simulink model "AcasXuClosedLoop_all_comp2017b"  (Simulink 9.0)
settings: Solver=VariableStepAuto  StartTime=0.0  StopTime=20  RelTol=1e-3
libraries: neural x14040, simulink x2
(part 30 of 534)

== system AcasXuClosedLoop_all_comp2017b/networks/Networks_m_7/AcasXu_3_7/Layer 3 ==
  "a{2} " : Inport  PortDimensions=50
      mask Display: e = 0.06;c1 = [120 230 180]/255;c2 = ([120 230 180]+0)/(256+128);c3 = ([120 230 180]+128)/(256+128);patch([0 1 1 0],[0 0 1 1],c1);patch([0 1 1 1-e 1-e 0+e],[0 0 1 1-e 0+e 0+e],c2);patch([0 0 1 1-e 0+e 0+e],[0 1 1 1-e 1-e 0+e],c3);plot([0 1 1 0 0],[0 0 1 1 0]);
  Delays 1 : Subsystem
      mask Display: e = 0.06;c1 = [120 150 230]/255;c2 = ([120 150 230]+0)/383;c3 = ([120 150 230]+128)/383;patch([0 1 1 0],[0 0 1 1],c1);patch([0 1 1 1-e 1-e 0+e],[0 0 1 1-e 0+e 0+e],c2);patch([0 0 1 1-e 0+e 0+e],[0 1 1 1-e 1-e 0+e],c3);plot([0 1 1 0 0],[0 0 1 1 0]);disp('0');
  "LW{3,2}" : Subsystem
      mask Display: e = 0.06;c1 = [120 150 230]/255;c2 = ([120 150 230]+0)/383;c3 = ([120 150 230]+128)/383;patch([0 1 1 0],[0 0 1 1],c1);patch([0 1 1 1-e 1-e 0+e],[0 0 1 1-e 0+e 0+e],c2);patch([0 0 1 1-e 0+e 0+e],[0 1 1 1-e 1-e 0+e],c3);plot([0 1 1 0 0],[0 0 1 1 0]);disp('W');
  "b{3}" : Constant  Value=[-0.5160109999999999974562570059788413345813751220703125;-1.036350000000000104449782156734727323055267333984375;-0.1930449999999999943778306032982072792947292327880859375;-1.3833800000000000540723021913436241447925567626953125;-0.9235130000000000283222334473975934088230133056640625;-1.2440800000000000746780415283865295350551605224609375;0.7431229999999999780158077555825002491474151611328125;-0.64134700000000000041922021409845910966396331787109375;-1.583579999999999987636556397774256765842437744140625;-0.76183800000000001517719283583573997020721435546875;-0.2049209999999999920472504300050786696374416351318359375;-1.2468999999999998973265746826655231416225433349609375;-0.497601000000000015521806062679388560354709625244140625;-1.144530000000000047322146201622672379016876220703125;1.0850699999999999789679350215010344982147216796875;-0.288870000000000015649703755116206593811511993408203125;-2.07702000000000008839151632855646312236785888671875;-0.65424599999999999422328755827038548886775970458984375;-1.87901000000000006906475391588173806667327880859375;-1.623250000000000081712414612411521375179290771484375;-0.0298465000000000015012435738981366739608347415924072265625;-1.91349999999999997868371792719699442386627197265625;-0.6310480000000000533333377461531199514865875244140625;-0.00068311999999999995121513496343368387897498905658721923828125;-1.6211100000000000509459141539991833269596099853515625;-1.2762800000000000810729261502274312078952789306640625;-0.61060099999999994935251379502005875110626220703125;-0.5314229999999999787263504913426004350185394287109375;-0.2066989999999999938484762651569326408207416534423828125;0.50820399999999998907895815136726014316082000732421875;-1.0196799999999999197797251326846890151500701904296875;-2.289410000000000167119651450775563716888427734375;0.65037800000000001166000629382324405014514923095703125;-1.8272999999999999243271986415493302047252655029296875;-1.99828999999999989967136571067385375499725341796875;-1.2788999999999999257482841130695305764675140380859375;-2.06318000000000001392663762089796364307403564453125;-0.86935200000000001363531509923632256686687469482421875;-2.26323000000000007503331289626657962799072265625;-0.0320645999999999986584953148849308490753173828125;-0.3529809999999999892139612711616791784763336181640625;-1.2028399999999999092636926434352062642574310302734375;-0.513816999999999968196107147377915680408477783203125;-0.6795139999999999513846660192939452826976776123046875;0.144722999999999990539123473354266025125980377197265625;0.28890300000000002089706185870454646646976470947265625;-0.7935410000000000518838305652025155723094940185546875;-0.295134999999999980691001155719277448952198028564453125;0.68889999999999995683452880257391370832920074462890625;-0.305371000000000003549160965121700428426265716552734375]
      mask Display: e = 0.06;c1 = [120 150 230]/255;c2 = ([120 150 230]+0)/383;c3 = ([120 150 230]+128)/383;patch([0 1 1 0],[0 0 1 1],c1);patch([0 1 1 1-e 1-e 0+e],[0 0 1 1-e 0+e 0+e],c2);patch([0 0 1 1-e 0+e 0+e],[0 1 1 1-e 1-e 0+e],c3);plot([0 1 1 0 0],[0 0 1 1 0]);disp('b');
  netsum : Sum  Inputs=2  SaturateOnIntegerOverflow=on  InputSameDT=on  OutDataTypeStr=Inherit: Same as first input
      mask Display: e = 0.06;c1 = [120 150 230]/255;c2 = ([120 150 230]+0)/383;c3 = ([120 150 230]+128)/383;patch([0 1 1 0],[0 0 1 1],c1);patch([0 1 1 1-e 1-e 0+e],[0 0 1 1-e 0+e 0+e],c2);patch([0 0 1 1-e 0+e 0+e],[0 1 1 1-e 1-e 0+e],c3);plot([0 1 1 0 0],[0 0 1 1 0]);disp('+');
  poslin : neural/Transfer Functions/poslin
      settings: SourceProductName=Deep Learning Toolbox  SourceProductBaseCode=NN
  "a{3}" : Outport  InitialOutput=[0;0;0;0;0;0;0;0;0;0;0;0;0;0;0;0;0;0;0;0;0;0;0;0;0;0;0;0;0;0;0;0;0;0;0;0;0;0;0;0;0;0;0;0;0;0;0;0;0;0]
      mask Display: e = 0.06;c1 = [120 230 180]/255;c2 = ([120 230 180]+0)/(256+128);c3 = ([120 230 180]+128)/(256+128);patch([0 1 1 0],[0 0 1 1],c1);patch([0 1 1 1-e 1-e 0+e],[0 0 1 1-e 0+e 0+e],c2);patch([0 0 1 1-e 0+e 0+e],[0 1 1 1-e 1-e 0+e],c3);plot([0 1 1 0 0],[0 0 1 1 0]);
  wires:
  poslin/1 -> "a{3}"/1
  netsum/1 -> poslin/1
  "b{3}"/1 -> netsum/2
  "LW{3,2}"/1("lz{3,2}") -> netsum/1
  Delays 1/1("pd{3,2}") -> "LW{3,2}"/1("ad{3,2}")
  "a{2} "/1 -> Delays 1/1("p{3}")

== system AcasXuClosedLoop_all_comp2017b/networks/Networks_m_7/AcasXu_3_7/Layer 4 ==
  "a{3} " : Inport  PortDimensions=50
      mask Display: e = 0.06;c1 = [120 230 180]/255;c2 = ([120 230 180]+0)/(256+128);c3 = ([120 230 180]+128)/(256+128);patch([0 1 1 0],[0 0 1 1],c1);patch([0 1 1 1-e 1-e 0+e],[0 0 1 1-e 0+e 0+e],c2);patch([0 0 1 1-e 0+e 0+e],[0 1 1 1-e 1-e 0+e],c3);plot([0 1 1 0 0],[0 0 1 1 0]);
  Delays 1 : Subsystem
      mask Display: e = 0.06;c1 = [120 150 230]/255;c2 = ([120 150 230]+0)/383;c3 = ([120 150 230]+128)/383;patch([0 1 1 0],[0 0 1 1],c1);patch([0 1 1 1-e 1-e 0+e],[0 0 1 1-e 0+e 0+e],c2);patch([0 0 1 1-e 0+e 0+e],[0 1 1 1-e 1-e 0+e],c3);plot([0 1 1 0 0],[0 0 1 1 0]);disp('0');
  "LW{4,3}" : Subsystem
      mask Display: e = 0.06;c1 = [120 150 230]/255;c2 = ([120 150 230]+0)/383;c3 = ([120 150 230]+128)/383;patch([0 1 1 0],[0 0 1 1],c1);patch([0 1 1 1-e 1-e 0+e],[0 0 1 1-e 0+e 0+e],c2);patch([0 0 1 1-e 0+e 0+e],[0 1 1 1-e 1-e 0+e],c3);plot([0 1 1 0 0],[0 0 1 1 0]);disp('W');
  "b{4}" : Constant  Value=[-2.0283400000000000318323145620524883270263671875;-0.91175300000000003564082362572662532329559326171875;-0.73282999999999998141930745987338013947010040283203125;-0.789271999999999973596231939154677093029022216796875;-0.158094000000000012295942042328533716499805450439453125;0.2696600000000000108002495835535228252410888671875;1.2570099999999999607069867124664597213268280029296875;-0.82799900000000004052225222039851360023021697998046875;3.14252000000000020207835405017249286174774169921875;2.064109999999999889297441768576391041278839111328125;-0.74948499999999995679900166578590869903564453125;0.464484999999999981223908207539352588355541229248046875;0.52677700000000005076117304270155727863311767578125;-0.5944760000000000044195758164278231561183929443359375;0.120389999999999997015720509807579219341278076171875;0.376414999999999999591437926937942393124103546142578125;0.5400139999999999940172301648999564349651336669921875;1.5614699999999999135980033315718173980712890625;0.284804000000000001602273869139025919139385223388671875;1.98913999999999990819787853979505598545074462890625;-8.2001200000000000756017470848746597766876220703125;1.5404999999999999804600747665972448885440826416015625;2.850919999999999898676605880609713494777679443359375;0.2247550000000000103295150211124564521014690399169921875;0.038227799999999999280841933568808599375188350677490234375;-0.12055100000000000537436761760545778088271617889404296875;-0.050302199999999998303312764846850768662989139556884765625;2.07948999999999983856469043530523777008056640625;-2.65962999999999993860910763032734394073486328125;2.894480000000000163851154866279102861881256103515625;1.542359999999999953246287986985407769680023193359375;3.62826000000000004064304448547773063182830810546875;-0.008326540000000000329993810055384528823196887969970703125;2.3668800000000000949285094975493848323822021484375;-5.91856999999999988659737937268801033496856689453125;1.177869999999999972573050399660132825374603271484375;3.14069000000000020378365661599673330783843994140625;-0.443124000000000017873702518045320175588130950927734375;-1.71642999999999990023979989928193390369415283203125;0.70271799999999995378630046616308391094207763671875;2.805610000000000159303681357414461672306060791015625;0.153108999999999995100807836934109218418598175048828125;0.213351000000000012857270803579012863337993621826171875;0.1404829999999999967652541954521439038217067718505859375;-3.570650000000000101607611213694326579570770263671875;3.355370000000000185735871127690188586711883544921875;0.985071000000000029928060030215419828891754150390625;2.59541000000000021685764295398257672786712646484375;1.6361200000000000187583282240666449069976806640625;3.28422999999999998266275724745355546474456787109375]
      mask Display: e = 0.06;c1 = [120 150 230]/255;c2 = ([120 150 230]+0)/383;c3 = ([120 150 230]+128)/383;patch([0 1 1 0],[0 0 1 1],c1);patch([0 1 1 1-e 1-e 0+e],[0 0 1 1-e 0+e 0+e],c2);patch([0 0 1 1-e 0+e 0+e],[0 1 1 1-e 1-e 0+e],c3);plot([0 1 1 0 0],[0 0 1 1 0]);disp('b');
  netsum : Sum  Inputs=2  SaturateOnIntegerOverflow=on  InputSameDT=on  OutDataTypeStr=Inherit: Same as first input
      mask Display: e = 0.06;c1 = [120 150 230]/255;c2 = ([120 150 230]+0)/383;c3 = ([120 150 230]+128)/383;patch([0 1 1 0],[0 0 1 1],c1);patch([0 1 1 1-e 1-e 0+e],[0 0 1 1-e 0+e 0+e],c2);patch([0 0 1 1-e 0+e 0+e],[0 1 1 1-e 1-e 0+e],c3);plot([0 1 1 0 0],[0 0 1 1 0]);disp('+');
  poslin : neural/Transfer Functions/poslin
      settings: SourceProductName=Deep Learning Toolbox  SourceProductBaseCode=NN
  "a{4}" : Outport  InitialOutput=[0;0;0;0;0;0;0;0;0;0;0;0;0;0;0;0;0;0;0;0;0;0;0;0;0;0;0;0;0;0;0;0;0;0;0;0;0;0;0;0;0;0;0;0;0;0;0;0;0;0]
      mask Display: e = 0.06;c1 = [120 230 180]/255;c2 = ([120 230 180]+0)/(256+128);c3 = ([120 230 180]+128)/(256+128);patch([0 1 1 0],[0 0 1 1],c1);patch([0 1 1 1-e 1-e 0+e],[0 0 1 1-e 0+e 0+e],c2);patch([0 0 1 1-e 0+e 0+e],[0 1 1 1-e 1-e 0+e],c3);plot([0 1 1 0 0],[0 0 1 1 0]);
  wires:
  poslin/1 -> "a{4}"/1
  netsum/1 -> poslin/1
  "b{4}"/1 -> netsum/2
  "LW{4,3}"/1("lz{4,3}") -> netsum/1
  Delays 1/1("pd{4,3}") -> "LW{4,3}"/1("ad{4,3}")
  "a{3} "/1 -> Delays 1/1("p{4}")

== system AcasXuClosedLoop_all_comp2017b/networks/Networks_m_7/AcasXu_3_7/Layer 5 ==
  "a{4} " : Inport  PortDimensions=50
      mask Display: e = 0.06;c1 = [120 230 180]/255;c2 = ([120 230 180]+0)/(256+128);c3 = ([120 230 180]+128)/(256+128);patch([0 1 1 0],[0 0 1 1],c1);patch([0 1 1 1-e 1-e 0+e],[0 0 1 1-e 0+e 0+e],c2);patch([0 0 1 1-e 0+e 0+e],[0 1 1 1-e 1-e 0+e],c3);plot([0 1 1 0 0],[0 0 1 1 0]);
  Delays 1 : Subsystem
      mask Display: e = 0.06;c1 = [120 150 230]/255;c2 = ([120 150 230]+0)/383;c3 = ([120 150 230]+128)/383;patch([0 1 1 0],[0 0 1 1],c1);patch([0 1 1 1-e 1-e 0+e],[0 0 1 1-e 0+e 0+e],c2);patch([0 0 1 1-e 0+e 0+e],[0 1 1 1-e 1-e 0+e],c3);plot([0 1 1 0 0],[0 0 1 1 0]);disp('0');
  "LW{5,4}" : Subsystem
      mask Display: e = 0.06;c1 = [120 150 230]/255;c2 = ([120 150 230]+0)/383;c3 = ([120 150 230]+128)/383;patch([0 1 1 0],[0 0 1 1],c1);patch([0 1 1 1-e 1-e 0+e],[0 0 1 1-e 0+e 0+e],c2);patch([0 0 1 1-e 0+e 0+e],[0 1 1 1-e 1-e 0+e],c3);plot([0 1 1 0 0],[0 0 1 1 0]);disp('W');
  "b{5}" : Constant  Value=[-1.831739999999999923829818726517260074615478515625;0.480563000000000017930545936906128190457820892333984375;-1.908349999999999990762944435118697583675384521484375;-0.18985099999999999198507794062606990337371826171875;-1.503670000000000062101435105432756245136260986328125;-0.111736000000000001985966946449480019509792327880859375;-1.2899499999999999300115405276301316916942596435546875;-0.724744999999999972573050399660132825374603271484375;-1.0682700000000000528643795405514538288116455078125;2.181929999999999925108795650885440409183502197265625;0.117064000000000001389111048410995863378047943115234375;1.7841199999999999281641294146538712084293365478515625;-2.4140899999999998470912032644264400005340576171875;1.2328699999999999104005610206513665616512298583984375;-0.387774000000000007570832849523867480456829071044921875;-1.338649999999999895550217843265272676944732666015625;-2.837140000000000217283968595438636839389801025390625;-0.94584900000000005082512188891996629536151885986328125;1.0748200000000001086419842977193184196949005126953125;-0.90491800000000000014921397450962103903293609619140625;-0.309447000000000027597479856922291219234466552734375;1.360349999999999948130380289512686431407928466796875;-0.9989630000000000453752591056399978697299957275390625;0.4987880000000000091375795818748883903026580810546875;2.24645999999999990137666827649809420108795166015625;-2.6241099999999999425881469505839049816131591796875;-0.87940399999999996349941966400365345180034637451171875;2.75837999999999983202769726631231606006622314453125;0.333708999999999977870146494751679711043834686279296875;2.07085000000000007958078640513122081756591796875;-1.1539200000000000567723645872320048511028289794921875;0.28667399999999998438937609535059891641139984130859375;-2.287739999999999884749968259711749851703643798828125;-1.3585499999999999243271986415493302047252655029296875;-2.09518000000000004234834705130197107791900634765625;-0.03333239999999999814139783893551793880760669708251953125;-1.2466299999999999048583276817225851118564605712890625;2.039960000000000217568185689742676913738250732421875;1.325229999999999908055769992643035948276519775390625;-2.01517999999999997129407347529195249080657958984375;-0.9651009999999999866560074224253185093402862548828125;-0.429591999999999973880449033458717167377471923828125;2.677569999999999783568682687473483383655548095703125;-2.484420000000000072759576141834259033203125;0.483877999999999974800601876268046908080577850341796875;-1.314319999999999932782657197094522416591644287109375;4.995779999999999887450030655600130558013916015625;-3.328160000000000007247535904753021895885467529296875;-4.19460999999999994969357430818490684032440185546875;-0.81477699999999997348965052879066206514835357666015625]
      mask Display: e = 0.06;c1 = [120 150 230]/255;c2 = ([120 150 230]+0)/383;c3 = ([120 150 230]+128)/383;patch([0 1 1 0],[0 0 1 1],c1);patch([0 1 1 1-e 1-e 0+e],[0 0 1 1-e 0+e 0+e],c2);patch([0 0 1 1-e 0+e 0+e],[0 1 1 1-e 1-e 0+e],c3);plot([0 1 1 0 0],[0 0 1 1 0]);disp('b');
  netsum : Sum  Inputs=2  SaturateOnIntegerOverflow=on  InputSameDT=on  OutDataTypeStr=Inherit: Same as first input
      mask Display: e = 0.06;c1 = [120 150 230]/255;c2 = ([120 150 230]+0)/383;c3 = ([120 150 230]+128)/383;patch([0 1 1 0],[0 0 1 1],c1);patch([0 1 1 1-e 1-e 0+e],[0 0 1 1-e 0+e 0+e],c2);patch([0 0 1 1-e 0+e 0+e],[0 1 1 1-e 1-e 0+e],c3);plot([0 1 1 0 0],[0 0 1 1 0]);disp('+');
  poslin : neural/Transfer Functions/poslin
      settings: SourceProductName=Deep Learning Toolbox  SourceProductBaseCode=NN
  "a{5}" : Outport  InitialOutput=[0;0;0;0;0;0;0;0;0;0;0;0;0;0;0;0;0;0;0;0;0;0;0;0;0;0;0;0;0;0;0;0;0;0;0;0;0;0;0;0;0;0;0;0;0;0;0;0;0;0]
      mask Display: e = 0.06;c1 = [120 230 180]/255;c2 = ([120 230 180]+0)/(256+128);c3 = ([120 230 180]+128)/(256+128);patch([0 1 1 0],[0 0 1 1],c1);patch([0 1 1 1-e 1-e 0+e],[0 0 1 1-e 0+e 0+e],c2);patch([0 0 1 1-e 0+e 0+e],[0 1 1 1-e 1-e 0+e],c3);plot([0 1 1 0 0],[0 0 1 1 0]);
  wires:
  poslin/1 -> "a{5}"/1
  netsum/1 -> poslin/1
  "b{5}"/1 -> netsum/2
  "LW{5,4}"/1("lz{5,4}") -> netsum/1
  Delays 1/1("pd{5,4}") -> "LW{5,4}"/1("ad{5,4}")
  "a{4} "/1 -> Delays 1/1("p{5}")

== system AcasXuClosedLoop_all_comp2017b/networks/Networks_m_7/AcasXu_3_7/Layer 6 ==
  "a{5} " : Inport  PortDimensions=50
      mask Display: e = 0.06;c1 = [120 230 180]/255;c2 = ([120 230 180]+0)/(256+128);c3 = ([120 230 180]+128)/(256+128);patch([0 1 1 0],[0 0 1 1],c1);patch([0 1 1 1-e 1-e 0+e],[0 0 1 1-e 0+e 0+e],c2);patch([0 0 1 1-e 0+e 0+e],[0 1 1 1-e 1-e 0+e],c3);plot([0 1 1 0 0],[0 0 1 1 0]);
  Delays 1 : Subsystem
      mask Display: e = 0.06;c1 = [120 150 230]/255;c2 = ([120 150 230]+0)/383;c3 = ([120 150 230]+128)/383;patch([0 1 1 0],[0 0 1 1],c1);patch([0 1 1 1-e 1-e 0+e],[0 0 1 1-e 0+e 0+e],c2);patch([0 0 1 1-e 0+e 0+e],[0 1 1 1-e 1-e 0+e],c3);plot([0 1 1 0 0],[0 0 1 1 0]);disp('0');
  "LW{6,5}" : Subsystem
      mask Display: e = 0.06;c1 = [120 150 230]/255;c2 = ([120 150 230]+0)/383;c3 = ([120 150 230]+128)/383;patch([0 1 1 0],[0 0 1 1],c1);patch([0 1 1 1-e 1-e 0+e],[0 0 1 1-e 0+e 0+e],c2);patch([0 0 1 1-e 0+e 0+e],[0 1 1 1-e 1-e 0+e],c3);plot([0 1 1 0 0],[0 0 1 1 0]);disp('W');
  "b{6}" : Constant  Value=[-4.73083999999999971208808347000740468502044677734375;-1.041349999999999997868371792719699442386627197265625;-9.7840900000000008418510333285667002201080322265625;-10.127700000000000812860889709554612636566162109375;-9.4367800000000006122036211309023201465606689453125;-7.17502999999999957481122692115604877471923828125;-8.1601300000000005496758603840135037899017333984375;4.4342600000000000903810359886847436428070068359375;-1.8521199999999999885602619542623870074748992919921875;0.68789000000000000145661260830820538103580474853515625;1.7980799999999999005950712671619839966297149658203125;-0.08598980000000000512017095388728193938732147216796875;-9.49371000000000009322320693172514438629150390625;0.43141200000000001768540869306889362633228302001953125;-2.422730000000000050164317144663073122501373291015625;-0.0302575999999999990797139304277152405120432376861572265625;0.73591499999999998582467242158600129187107086181640625;-2.705690000000000150492951433989219367504119873046875;0.338210999999999983867127184566925279796123504638671875;-3.032970000000000165840674526407383382320404052734375;0.898093999999999947903006614069454371929168701171875;-10.4324999999999992184029906638897955417633056640625;0.317664000000000001922018100231071002781391143798828125;-2.191050000000000164135371960583142936229705810546875;-0.152141999999999999459987520822323858737945556640625;-7.7293599999999997862687450833618640899658203125;-7.0383300000000001972466634470038115978240966796875;0.0738343999999999944794382145119016058743000030517578125;-1.0591399999999999703703679188038222491741180419921875;-0.162279000000000006576073019459727220237255096435546875;-0.166455999999999992855492791932192631065845489501953125;-1.8772200000000001107736125049996189773082733154296875;-0.4794359999999999732978039901354350149631500244140625;-2.790999999999999925393012745189480483531951904296875;0.370794000000000012473577726268558762967586517333984375;-5.665639999999999787405613460578024387359619140625;-6.6904500000000002302158463862724602222442626953125;0.36489599999999999813127260495093651115894317626953125;-2.462260000000000115250031740288250148296356201171875;-1.66561000000000003495870259939692914485931396484375;-1.1172999999999999598543354295543394982814788818359375;-9.5535599999999991638333085575141012668609619140625;0.397942000000000017934098650584928691387176513671875;-7.926520000000000010231815394945442676544189453125;-7.3880800000000004246203388902358710765838623046875;0.050423799999999997789590366892298334278166294097900390625;-0.76354500000000002923883357652812264859676361083984375;-1.6177799999999999958077978590154089033603668212890625;-5.27761999999999975585751599282957613468170166015625;0.53080899999999997529442907762131653726100921630859375]
      mask Display: e = 0.06;c1 = [120 150 230]/255;c2 = ([120 150 230]+0)/383;c3 = ([120 150 230]+128)/383;patch([0 1 1 0],[0 0 1 1],c1);patch([0 1 1 1-e 1-e 0+e],[0 0 1 1-e 0+e 0+e],c2);patch([0 0 1 1-e 0+e 0+e],[0 1 1 1-e 1-e 0+e],c3);plot([0 1 1 0 0],[0 0 1 1 0]);disp('b');
  netsum : Sum  Inputs=2  SaturateOnIntegerOverflow=on  InputSameDT=on  OutDataTypeStr=Inherit: Same as first input
      mask Display: e = 0.06;c1 = [120 150 230]/255;c2 = ([120 150 230]+0)/383;c3 = ([120 150 230]+128)/383;patch([0 1 1 0],[0 0 1 1],c1);patch([0 1 1 1-e 1-e 0+e],[0 0 1 1-e 0+e 0+e],c2);patch([0 0 1 1-e 0+e 0+e],[0 1 1 1-e 1-e 0+e],c3);plot([0 1 1 0 0],[0 0 1 1 0]);disp('+');
  poslin : neural/Transfer Functions/poslin
      settings: SourceProductName=Deep Learning Toolbox  SourceProductBaseCode=NN
  "a{6}" : Outport  InitialOutput=[0;0;0;0;0;0;0;0;0;0;0;0;0;0;0;0;0;0;0;0;0;0;0;0;0;0;0;0;0;0;0;0;0;0;0;0;0;0;0;0;0;0;0;0;0;0;0;0;0;0]
      mask Display: e = 0.06;c1 = [120 230 180]/255;c2 = ([120 230 180]+0)/(256+128);c3 = ([120 230 180]+128)/(256+128);patch([0 1 1 0],[0 0 1 1],c1);patch([0 1 1 1-e 1-e 0+e],[0 0 1 1-e 0+e 0+e],c2);patch([0 0 1 1-e 0+e 0+e],[0 1 1 1-e 1-e 0+e],c3);plot([0 1 1 0 0],[0 0 1 1 0]);
  wires:
  poslin/1 -> "a{6}"/1
  netsum/1 -> poslin/1
  "b{6}"/1 -> netsum/2
  "LW{6,5}"/1("lz{6,5}") -> netsum/1
  Delays 1/1("pd{6,5}") -> "LW{6,5}"/1("ad{6,5}")
  "a{5} "/1 -> Delays 1/1("p{6}")

== system AcasXuClosedLoop_all_comp2017b/networks/Networks_m_7/AcasXu_3_7/Layer 7 ==
  "a{6} " : Inport  PortDimensions=50
      mask Display: e = 0.06;c1 = [120 230 180]/255;c2 = ([120 230 180]+0)/(256+128);c3 = ([120 230 180]+128)/(256+128);patch([0 1 1 0],[0 0 1 1],c1);patch([0 1 1 1-e 1-e 0+e],[0 0 1 1-e 0+e 0+e],c2);patch([0 0 1 1-e 0+e 0+e],[0 1 1 1-e 1-e 0+e],c3);plot([0 1 1 0 0],[0 0 1 1 0]);
  Delays 1 : Subsystem
      mask Display: e = 0.06;c1 = [120 150 230]/255;c2 = ([120 150 230]+0)/383;c3 = ([120 150 230]+128)/383;patch([0 1 1 0],[0 0 1 1],c1);patch([0 1 1 1-e 1-e 0+e],[0 0 1 1-e 0+e 0+e],c2);patch([0 0 1 1-e 0+e 0+e],[0 1 1 1-e 1-e 0+e],c3);plot([0 1 1 0 0],[0 0 1 1 0]);disp('0');
  "LW{7,6}" : Subsystem
      mask Display: e = 0.06;c1 = [120 150 230]/255;c2 = ([120 150 230]+0)/383;c3 = ([120 150 230]+128)/383;patch([0 1 1 0],[0 0 1 1],c1);patch([0 1 1 1-e 1-e 0+e],[0 0 1 1-e 0+e 0+e],c2);patch([0 0 1 1-e 0+e 0+e],[0 1 1 1-e 1-e 0+e],c3);plot([0 1 1 0 0],[0 0 1 1 0]);disp('W');
  "b{7}" : Constant  Value=[-0.017999500000000001609379296496626920998096466064453125;0.020444600000000000272759592689908458851277828216552734375;-0.019109700000000000297273317073631915263831615447998046875;0.021079699999999999826538754632565542124211788177490234375;-0.0161877999999999987179588600838542333804070949554443359375]
      mask Display: e = 0.06;c1 = [120 150 230]/255;c2 = ([120 150 230]+0)/383;c3 = ([120 150 230]+128)/383;patch([0 1 1 0],[0 0 1 1],c1);patch([0 1 1 1-e 1-e 0+e],[0 0 1 1-e 0+e 0+e],c2);patch([0 0 1 1-e 0+e 0+e],[0 1 1 1-e 1-e 0+e],c3);plot([0 1 1 0 0],[0 0 1 1 0]);disp('b');
  netsum : Sum  Inputs=2  SaturateOnIntegerOverflow=on  InputSameDT=on  OutDataTypeStr=Inherit: Same as first input
      mask Display: e = 0.06;c1 = [120 150 230]/255;c2 = ([120 150 230]+0)/383;c3 = ([120 150 230]+128)/383;patch([0 1 1 0],[0 0 1 1],c1);patch([0 1 1 1-e 1-e 0+e],[0 0 1 1-e 0+e 0+e],c2);patch([0 0 1 1-e 0+e 0+e],[0 1 1 1-e 1-e 0+e],c3);plot([0 1 1 0 0],[0 0 1 1 0]);disp('+');
  purelin : neural/Transfer Functions/purelin
      settings: SourceProductName=Deep Learning Toolbox
  "a{7}" : Outport  InitialOutput=[0;0;0;0;0]
      mask Display: e = 0.06;c1 = [120 230 180]/255;c2 = ([120 230 180]+0)/(256+128);c3 = ([120 230 180]+128)/(256+128);patch([0 1 1 0],[0 0 1 1],c1);patch([0 1 1 1-e 1-e 0+e],[0 0 1 1-e 0+e 0+e],c2);patch([0 0 1 1-e 0+e 0+e],[0 1 1 1-e 1-e 0+e],c3);plot([0 1 1 0 0],[0 0 1 1 0]);
  wires:
  purelin/1 -> "a{7}"/1
  netsum/1 -> purelin/1
  "b{7}"/1 -> netsum/2
  "LW{7,6}"/1("lz{7,6}") -> netsum/1
  Delays 1/1("pd{7,6}") -> "LW{7,6}"/1("ad{7,6}")
  "a{6} "/1 -> Delays 1/1("p{7}")

== system AcasXuClosedLoop_all_comp2017b/networks/Networks_m_7/AcasXu_3_7/Process Input 1 ==
  x : Inport  PortDimensions=5
      mask Display: e = 0.06;c1 = [120 230 180]/255;c2 = ([120 230 180]+0)/(256+128);c3 = ([120 230 180]+128)/(256+128);patch([0 1 1 0],[0 0 1 1],c1);patch([0 1 1 1-e 1-e 0+e],[0 0 1 1-e 0+e 0+e],c2);patch([0 0 1 1-e 0+e 0+e],[0 1 1 1-e 1-e 0+e],c3);plot([0 1 1 0 0],[0 0 1 1 0]);
  p : Outport  PortDimensions=5
      mask Display: e = 0.06;c1 = [120 230 180]/255;c2 = ([120 230 180]+0)/(256+128);c3 = ([120 230 180]+128)/(256+128);patch([0 1 1 0],[0 0 1 1],c1);patch([0 1 1 1-e 1-e 0+e],[0 0 1 1-e 0+e 0+e],c2);patch([0 0 1 1-e 0+e 0+e],[0 1 1 1-e 1-e 0+e],c3);plot([0 1 1 0 0],[0 0 1 1 0]);
  wires:
  x/1 -> p/1

== system AcasXuClosedLoop_all_comp2017b/networks/Networks_m_7/AcasXu_3_7/Process Output 1 ==
  a : Inport  PortDimensions=5
      mask Display: e = 0.06;c1 = [120 230 180]/255;c2 = ([120 230 180]+0)/(256+128);c3 = ([120 230 180]+128)/(256+128);patch([0 1 1 0],[0 0 1 1],c1);patch([0 1 1 1-e 1-e 0+e],[0 0 1 1-e 0+e 0+e],c2);patch([0 0 1 1-e 0+e 0+e],[0 1 1 1-e 1-e 0+e],c3);plot([0 1 1 0 0],[0 0 1 1 0]);
  y : Outport  PortDimensions=5
      mask Display: e = 0.06;c1 = [120 230 180]/255;c2 = ([120 230 180]+0)/(256+128);c3 = ([120 230 180]+128)/(256+128);patch([0 1 1 0],[0 0 1 1],c1);patch([0 1 1 1-e 1-e 0+e],[0 0 1 1-e 0+e 0+e],c2);patch([0 0 1 1-e 0+e 0+e],[0 1 1 1-e 1-e 0+e],c3);plot([0 1 1 0 0],[0 0 1 1 0]);
  wires:
  a/1 -> y/1

== system AcasXuClosedLoop_all_comp2017b/networks/Networks_m_7/AcasXu_4_7/Layer 1 ==
  "p{1}" : Inport  PortDimensions=5
      mask Display: e = 0.06;c1 = [120 230 180]/255;c2 = ([120 230 180]+0)/(256+128);c3 = ([120 230 180]+128)/(256+128);patch([0 1 1 0],[0 0 1 1],c1);patch([0 1 1 1-e 1-e 0+e],[0 0 1 1-e 0+e 0+e],c2);patch([0 0 1 1-e 0+e 0+e],[0 1 1 1-e 1-e 0+e],c3);plot([0 1 1 0 0],[0 0 1 1 0]);
  Delays 1 : Subsystem
      mask Display: e = 0.06;c1 = [120 150 230]/255;c2 = ([120 150 230]+0)/383;c3 = ([120 150 230]+128)/383;patch([0 1 1 0],[0 0 1 1],c1);patch([0 1 1 1-e 1-e 0+e],[0 0 1 1-e 0+e 0+e],c2);patch([0 0 1 1-e 0+e 0+e],[0 1 1 1-e 1-e 0+e],c3);plot([0 1 1 0 0],[0 0 1 1 0]);disp('0');
  "IW{1,1}" : Subsystem
      mask Display: e = 0.06;c1 = [120 150 230]/255;c2 = ([120 150 230]+0)/383;c3 = ([120 150 230]+128)/383;patch([0 1 1 0],[0 0 1 1],c1);patch([0 1 1 1-e 1-e 0+e],[0 0 1 1-e 0+e 0+e],c2);patch([0 0 1 1-e 0+e 0+e],[0 1 1 1-e 1-e 0+e],c3);plot([0 1 1 0 0],[0 0 1 1 0]);disp('W');
  "b{1}" : Constant  Value=[-0.374502999999999974800601876268046908080577850341796875;-0.37704199999999998826893943260074593126773834228515625;-0.3557799999999999851496568226139061152935028076171875;-0.1670959999999999945341500051654293201863765716552734375;0.91608599999999995588950696401298046112060546875;-0.207760000000000000230926389122032560408115386962890625;-0.50561599999999995436184008212876506149768829345703125;0.1281339999999999978985698589895037002861499786376953125;-0.1014710000000000056363802514169947244226932525634765625;-0.05787520000000000164330771212917170487344264984130859375;0.1384869999999999989892529583812574855983257293701171875;0.0715102999999999988656185223590000532567501068115234375;0.028768200000000000715605352752390899695456027984619140625;-0.007550659999999999837105857380947782075963914394378662109375;0.1515559999999999962749797077776747755706310272216796875;-0.01397499999999999957533969308087762328796088695526123046875;-0.020845200000000001228084300919363158755004405975341796875;0.163808000000000009155343150268890894949436187744140625;0.28126499999999998724575789310620166361331939697265625;0.218264999999999986801668683256139047443866729736328125;0.172860999999999986886933811547351069748401641845703125;-0.32658500000000001417532757841399870812892913818359375;-0.0181639000000000001844302488507310044951736927032470703125;-0.35100399999999998268407352952635847032070159912109375;-0.058976599999999997081534530707358499057590961456298828125;0.0967816999999999982851051072429982014000415802001953125;-0.0262422999999999996545430036576362908817827701568603515625;0.10803300000000000402611277650066767819225788116455078125;0.071492100000000002868461024263524450361728668212890625;-0.56155999999999994809485315272468142211437225341796875;0.4309600000000000097344354799133725464344024658203125;0.01430720000000000081963325015976806753315031528472900390625;-0.0635450999999999932565941662687691859900951385498046875;0.06522150000000000169553260320753906853497028350830078125;-0.1759719999999999895390345727719250135123729705810546875;0.358677999999999996827426684831152670085430145263671875;0.2283139999999999891766577775342739187180995941162109375;-0.0275654000000000003189892794352999771945178508758544921875;-0.494981999999999977557507691017235629260540008544921875;-0.85107900000000002993516545757302083075046539306640625;-0.0136557999999999991891375117347706691361963748931884765625;-0.319272999999999973486097815111861564218997955322265625;-0.1507880000000000053628212981493561528623104095458984375;0.046815900000000000569233549185810261406004428863525390625;-0.27537800000000001166000629382324405014514923095703125;-0.58206199999999996830268855774193070828914642333984375;0.12407100000000000072919448257380281575024127960205078125;-0.3143029999999999990478727340814657509326934814453125;0.46144499999999999406696815640316344797611236572265625;-0.118387999999999993239185869242646731436252593994140625]
      mask Display: e = 0.06;c1 = [120 150 230]/255;c2 = ([120 150 230]+0)/383;c3 = ([120 150 230]+128)/383;patch([0 1 1 0],[0 0 1 1],c1);patch([0 1 1 1-e 1-e 0+e],[0 0 1 1-e 0+e 0+e],c2);patch([0 0 1 1-e 0+e 0+e],[0 1 1 1-e 1-e 0+e],c3);plot([0 1 1 0 0],[0 0 1 1 0]);disp('b');
  netsum : Sum  Inputs=2  SaturateOnIntegerOverflow=on  InputSameDT=on  OutDataTypeStr=Inherit: Same as first input
      mask Display: e = 0.06;c1 = [120 150 230]/255;c2 = ([120 150 230]+0)/383;c3 = ([120 150 230]+128)/383;patch([0 1 1 0],[0 0 1 1],c1);patch([0 1 1 1-e 1-e 0+e],[0 0 1 1-e 0+e 0+e],c2);patch([0 0 1 1-e 0+e 0+e],[0 1 1 1-e 1-e 0+e],c3);plot([0 1 1 0 0],[0 0 1 1 0]);disp('+');
  poslin : neural/Transfer Functions/poslin
      settings: SourceProductName=Deep Learning Toolbox  SourceProductBaseCode=NN
  "a{1}" : Outport  InitialOutput=[0;0;0;0;0;0;0;0;0;0;0;0;0;0;0;0;0;0;0;0;0;0;0;0;0;0;0;0;0;0;0;0;0;0;0;0;0;0;0;0;0;0;0;0;0;0;0;0;0;0]
      mask Display: e = 0.06;c1 = [120 230 180]/255;c2 = ([120 230 180]+0)/(256+128);c3 = ([120 230 180]+128)/(256+128);patch([0 1 1 0],[0 0 1 1],c1);patch([0 1 1 1-e 1-e 0+e],[0 0 1 1-e 0+e 0+e],c2);patch([0 0 1 1-e 0+e 0+e],[0 1 1 1-e 1-e 0+e],c3);plot([0 1 1 0 0],[0 0 1 1 0]);
  wires:
  poslin/1 -> "a{1}"/1
  netsum/1 -> poslin/1
  "b{1}"/1 -> netsum/2
  "IW{1,1}"/1("iz{1,1}") -> netsum/1
  Delays 1/1("pd{1,1}") -> "IW{1,1}"/1("pd{1,1}")
  "p{1}"/1 -> Delays 1/1("p{1}")

== system AcasXuClosedLoop_all_comp2017b/networks/Networks_m_7/AcasXu_4_7/Layer 2 ==
  "a{1} " : Inport  PortDimensions=50
      mask Display: e = 0.06;c1 = [120 230 180]/255;c2 = ([120 230 180]+0)/(256+128);c3 = ([120 230 180]+128)/(256+128);patch([0 1 1 0],[0 0 1 1],c1);patch([0 1 1 1-e 1-e 0+e],[0 0 1 1-e 0+e 0+e],c2);patch([0 0 1 1-e 0+e 0+e],[0 1 1 1-e 1-e 0+e],c3);plot([0 1 1 0 0],[0 0 1 1 0]);
  Delays 1 : Subsystem
      mask Display: e = 0.06;c1 = [120 150 230]/255;c2 = ([120 150 230]+0)/383;c3 = ([120 150 230]+128)/383;patch([0 1 1 0],[0 0 1 1],c1);patch([0 1 1 1-e 1-e 0+e],[0 0 1 1-e 0+e 0+e],c2);patch([0 0 1 1-e 0+e 0+e],[0 1 1 1-e 1-e 0+e],c3);plot([0 1 1 0 0],[0 0 1 1 0]);disp('0');
  "LW{2,1}" : Subsystem
      mask Display: e = 0.06;c1 = [120 150 230]/255;c2 = ([120 150 230]+0)/383;c3 = ([120 150 230]+128)/383;patch([0 1 1 0],[0 0 1 1],c1);patch([0 1 1 1-e 1-e 0+e],[0 0 1 1-e 0+e 0+e],c2);patch([0 0 1 1-e 0+e 0+e],[0 1 1 1-e 1-e 0+e],c3);plot([0 1 1 0 0],[0 0 1 1 0]);disp('W');
  "b{2}" : Constant  Value=[-0.0147247999999999996945110325441419263370335102081298828125;-0.10499999999999999611421941381195210851728916168212890625;-0.3082380000000000119797505249152891337871551513671875;-0.364308000000000020701662606370518915355205535888671875;-0.01410810000000000015429879596240425598807632923126220703125;0.53662200000000004340705572758452035486698150634765625;-0.01166080000000000067850169926941816811449825763702392578125;-0.032523000000000003295586026297314674593508243560791015625;0.5657929999999999903792513578082434833049774169921875;-0.0179296000000000003982147944725511479191482067108154296875;0.2150209999999999899156222227247781120240688323974609375;-0.73070800000000002416555844320100732147693634033203125;-0.390158000000000004803268893738277256488800048828125;-0.026139400000000000134914301952449022792279720306396484375;0.1867189999999999960866858828012482263147830963134765625;-0.0841625000000000011990408665951690636575222015380859375;1.18372000000000010544454198679886758327484130859375;-0.032603000000000000035971225997855071909725666046142578125;0.377495999999999998220090446920949034392833709716796875;1.1836999999999999744204615126363933086395263671875;-0.22695100000000001383426706524915061891078948974609375;-0.0209086999999999988142374007793478085659444332122802734375;-0.1897870000000000112461151502429856918752193450927734375;-1.0894999999999999129585148693877272307872772216796875;-0.66277799999999997826449771309853531420230865478515625;-1.0720799999999999219113533399649895727634429931640625;0.86568400000000000904520902622607536613941192626953125;-0.0107422999999999997655653061201519449241459369659423828125;0.022041100000000000969091473734806641004979610443115234375;0.07853290000000000270485855935476138256490230560302734375;0.003120340000000000092728047462742324569262564182281494140625;-0.01062980000000000001258992909924927516840398311614990234375;-0.0217696999999999994679367176786399795673787593841552734375;-0.1675780000000000047322146201622672379016876220703125;-0.01482849999999999966726615951984058483503758907318115234375;-0.01485700000000000041422421048764590523205697536468505859375;-0.022624999999999999278355033993648248724639415740966796875;-0.0113948000000000000675459688181945239193737506866455078125;0.1978040000000000075974782021148712374269962310791015625;-0.00943360000000000031850078130446490831673145294189453125;1.3995400000000000062527760746888816356658935546875;0.5653420000000000111839426608639769256114959716796875;-0.0845019999999999937845274189385236240923404693603515625;-1.6675599999999999312194631784223020076751708984375;0.769541999999999948300910546095110476016998291015625;-0.021421400000000000163336011382853030227124691009521484375;-0.403725999999999973777420336773502640426158905029296875;-1.1096399999999999597122268824023194611072540283203125;1.1998400000000000176214598468504846096038818359375;-1.6231400000000000272137867796118371188640594482421875]
      mask Display: e = 0.06;c1 = [120 150 230]/255;c2 = ([120 150 230]+0)/383;c3 = ([120 150 230]+128)/383;patch([0 1 1 0],[0 0 1 1],c1);patch([0 1 1 1-e 1-e 0+e],[0 0 1 1-e 0+e 0+e],c2);patch([0 0 1 1-e 0+e 0+e],[0 1 1 1-e 1-e 0+e],c3);plot([0 1 1 0 0],[0 0 1 1 0]);disp('b');
  netsum : Sum  Inputs=2  SaturateOnIntegerOverflow=on  InputSameDT=on  OutDataTypeStr=Inherit: Same as first input
      mask Display: e = 0.06;c1 = [120 150 230]/255;c2 = ([120 150 230]+0)/383;c3 = ([120 150 230]+128)/383;patch([0 1 1 0],[0 0 1 1],c1);patch([0 1 1 1-e 1-e 0+e],[0 0 1 1-e 0+e 0+e],c2);patch([0 0 1 1-e 0+e 0+e],[0 1 1 1-e 1-e 0+e],c3);plot([0 1 1 0 0],[0 0 1 1 0]);disp('+');
  poslin : neural/Transfer Functions/poslin
      settings: SourceProductName=Deep Learning Toolbox  SourceProductBaseCode=NN
  "a{2}" : Outport  InitialOutput=[0;0;0;0;0;0;0;0;0;0;0;0;0;0;0;0;0;0;0;0;0;0;0;0;0;0;0;0;0;0;0;0;0;0;0;0;0;0;0;0;0;0;0;0;0;0;0;0;0;0]
      mask Display: e = 0.06;c1 = [120 230 180]/255;c2 = ([120 230 180]+0)/(256+128);c3 = ([120 230 180]+128)/(256+128);patch([0 1 1 0],[0 0 1 1],c1);patch([0 1 1 1-e 1-e 0+e],[0 0 1 1-e 0+e 0+e],c2);patch([0 0 1 1-e 0+e 0+e],[0 1 1 1-e 1-e 0+e],c3);plot([0 1 1 0 0],[0 0 1 1 0]);
  wires:
  poslin/1 -> "a{2}"/1
  netsum/1 -> poslin/1
  "b{2}"/1 -> netsum/2
  "LW{2,1}"/1("lz{2,1}") -> netsum/1
  Delays 1/1("pd{2,1}") -> "LW{2,1}"/1("ad{2,1}")
  "a{1} "/1 -> Delays 1/1("p{2}")

== system AcasXuClosedLoop_all_comp2017b/networks/Networks_m_7/AcasXu_4_7/Layer 3 ==
  "a{2} " : Inport  PortDimensions=50
      mask Display: e = 0.06;c1 = [120 230 180]/255;c2 = ([120 230 180]+0)/(256+128);c3 = ([120 230 180]+128)/(256+128);patch([0 1 1 0],[0 0 1 1],c1);patch([0 1 1 1-e 1-e 0+e],[0 0 1 1-e 0+e 0+e],c2);patch([0 0 1 1-e 0+e 0+e],[0 1 1 1-e 1-e 0+e],c3);plot([0 1 1 0 0],[0 0 1 1 0]);
  Delays 1 : Subsystem
      mask Display: e = 0.06;c1 = [120 150 230]/255;c2 = ([120 150 230]+0)/383;c3 = ([120 150 230]+128)/383;patch([0 1 1 0],[0 0 1 1],c1);patch([0 1 1 1-e 1-e 0+e],[0 0 1 1-e 0+e 0+e],c2);patch([0 0 1 1-e 0+e 0+e],[0 1 1 1-e 1-e 0+e],c3);plot([0 1 1 0 0],[0 0 1 1 0]);disp('0');
  "LW{3,2}" : Subsystem
      mask Display: e = 0.06;c1 = [120 150 230]/255;c2 = ([120 150 230]+0)/383;c3 = ([120 150 230]+128)/383;patch([0 1 1 0],[0 0 1 1],c1);patch([0 1 1 1-e 1-e 0+e],[0 0 1 1-e 0+e 0+e],c2);patch([0 0 1 1-e 0+e 0+e],[0 1 1 1-e 1-e 0+e],c3);plot([0 1 1 0 0],[0 0 1 1 0]);disp('W');
  "b{3}" : Constant  Value=[1.476259999999999905639924691058695316314697265625;0.6009889999999999954383156364201568067073822021484375;-0.1485350000000000003641531520770513452589511871337890625;0.1516780000000000072635231163076241500675678253173828125;-0.48529699999999997839239540553535334765911102294921875;-2.484109999999999818243168192566372454166412353515625;-1.30675000000000007815970093361102044582366943359375;-1.130409999999999914876980255939997732639312744140625;-0.369945000000000023820945216357358731329441070556640625;0.56203999999999998404831558218575082719326019287109375;-0.522178999999999948755657896981574594974517822265625;0.563401999999999958390617393888533115386962890625;-0.021003500000000001335376254019138286821544170379638671875;0.342328000000000021163515384614584036171436309814453125;-0.892954000000000025494273359072394669055938720703125;-0.08194500000000000394795307556705665774643421173095703125;-0.68201599999999995560528986970894038677215576171875;-0.191946000000000005503153488461975939571857452392578125;-0.74880500000000005389466650740359909832477569580078125;-2.008509999999999795505800648243166506290435791015625;0.43734499999999998376409848788171075284481048583984375;-0.257222000000000006192379942149273119866847991943359375;0.76004400000000005288569582262425683438777923583984375;0.380220999999999975660358586537768132984638214111328125;0.09082020000000000370388164583346224389970302581787109375;0.628427999999999986613374858279712498188018798828125;-1.9202600000000000779465381128829903900623321533203125;-0.66152200000000005442046813186607323586940765380859375;-2.50349999999999983657517077517695724964141845703125;-0.9993760000000000420783408117131330072879791259765625;0.01576589999999999935909045234438963234424591064453125;0.46218199999999998173194626360782422125339508056640625;-0.67592300000000005155698090675286948680877685546875;0.545938000000000034361846701358444988727569580078125;-0.94438699999999997647393001898308284580707550048828125;-0.68770299999999995321786627755500376224517822265625;-0.60755999999999998895106045893044210970401763916015625;-0.25267899999999998694732994408695958554744720458984375;-0.46701599999999998669153455921332351863384246826171875;-0.5729729999999999545678974754991941154003143310546875;0.8004320000000000323581161865149624645709991455078125;-1.0569800000000000306243919112603180110454559326171875;-1.0081100000000000616040551904006861150264739990234375;-2.0118700000000000471800376544706523418426513671875;0.06494949999999999334931999328546226024627685546875;1.138149999999999995026200849679298698902130126953125;0.85822699999999996212096675662905909121036529541015625;1.023660000000000014352963262354023754596710205078125;-0.369491000000000013869794202037155628204345703125;-0.19858799999999998675548340543173253536224365234375]
      mask Display: e = 0.06;c1 = [120 150 230]/255;c2 = ([120 150 230]+0)/383;c3 = ([120 150 230]+128)/383;patch([0 1 1 0],[0 0 1 1],c1);patch([0 1 1 1-e 1-e 0+e],[0 0 1 1-e 0+e 0+e],c2);patch([0 0 1 1-e 0+e 0+e],[0 1 1 1-e 1-e 0+e],c3);plot([0 1 1 0 0],[0 0 1 1 0]);disp('b');
  netsum : Sum  Inputs=2  SaturateOnIntegerOverflow=on  InputSameDT=on  OutDataTypeStr=Inherit: Same as first input
      mask Display: e = 0.06;c1 = [120 150 230]/255;c2 = ([120 150 230]+0)/383;c3 = ([120 150 230]+128)/383;patch([0 1 1 0],[0 0 1 1],c1);patch([0 1 1 1-e 1-e 0+e],[0 0 1 1-e 0+e 0+e],c2);patch([0 0 1 1-e 0+e 0+e],[0 1 1 1-e 1-e 0+e],c3);plot([0 1 1 0 0],[0 0 1 1 0]);disp('+');
  poslin : neural/Transfer Functions/poslin
      settings: SourceProductName=Deep Learning Toolbox  SourceProductBaseCode=NN
  "a{3}" : Outport  InitialOutput=[0;0;0;0;0;0;0;0;0;0;0;0;0;0;0;0;0;0;0;0;0;0;0;0;0;0;0;0;0;0;0;0;0;0;0;0;0;0;0;0;0;0;0;0;0;0;0;0;0;0]
      mask Display: e = 0.06;c1 = [120 230 180]/255;c2 = ([120 230 180]+0)/(256+128);c3 = ([120 230 180]+128)/(256+128);patch([0 1 1 0],[0 0 1 1],c1);patch([0 1 1 1-e 1-e 0+e],[0 0 1 1-e 0+e 0+e],c2);patch([0 0 1 1-e 0+e 0+e],[0 1 1 1-e 1-e 0+e],c3);plot([0 1 1 0 0],[0 0 1 1 0]);
  wires:
  poslin/1 -> "a{3}"/1
  netsum/1 -> poslin/1
  "b{3}"/1 -> netsum/2
  "LW{3,2}"/1("lz{3,2}") -> netsum/1
  Delays 1/1("pd{3,2}") -> "LW{3,2}"/1("ad{3,2}")
  "a{2} "/1 -> Delays 1/1("p{3}")

== system AcasXuClosedLoop_all_comp2017b/networks/Networks_m_7/AcasXu_4_7/Layer 4 ==
  "a{3} " : Inport  PortDimensions=50
      mask Display: e = 0.06;c1 = [120 230 180]/255;c2 = ([120 230 180]+0)/(256+128);c3 = ([120 230 180]+128)/(256+128);patch([0 1 1 0],[0 0 1 1],c1);patch([0 1 1 1-e 1-e 0+e],[0 0 1 1-e 0+e 0+e],c2);patch([0 0 1 1-e 0+e 0+e],[0 1 1 1-e 1-e 0+e],c3);plot([0 1 1 0 0],[0 0 1 1 0]);
  Delays 1 : Subsystem
      mask Display: e = 0.06;c1 = [120 150 230]/255;c2 = ([120 150 230]+0)/383;c3 = ([120 150 230]+128)/383;patch([0 1 1 0],[0 0 1 1],c1);patch([0 1 1 1-e 1-e 0+e],[0 0 1 1-e 0+e 0+e],c2);patch([0 0 1 1-e 0+e 0+e],[0 1 1 1-e 1-e 0+e],c3);plot([0 1 1 0 0],[0 0 1 1 0]);disp('0');
  "LW{4,3}" : Subsystem
      mask Display: e = 0.06;c1 = [120 150 230]/255;c2 = ([120 150 230]+0)/383;c3 = ([120 150 230]+128)/383;patch([0 1 1 0],[0 0 1 1],c1);patch([0 1 1 1-e 1-e 0+e],[0 0 1 1-e 0+e 0+e],c2);patch([0 0 1 1-e 0+e 0+e],[0 1 1 1-e 1-e 0+e],c3);plot([0 1 1 0 0],[0 0 1 1 0]);disp('W');
  "b{4}" : Constant  Value=[-2.15674000000000010146550266654230654239654541015625;-3.224950000000000205346850634668953716754913330078125;-1.020329999999999959214846967370249330997467041015625;0.95015400000000005409361847341642715036869049072265625;0.0840506000000000030869529155097552575170993804931640625;-0.0186541999999999992543298077407598611898720264434814453125;0.293300999999999978395948119214153848588466644287109375;-1.0007200000000000539301936441916041076183319091796875;1.7944500000000001005417971100541763007640838623046875;-1.1884799999999999808864004080533050000667572021484375;-0.02617490000000000094360075308941304683685302734375;0.462901999999999980150988676541601307690143585205078125;0.0826450999999999991185717362895957194268703460693359375;-0.4663880000000000247695197685970924794673919677734375;-2.290810000000000012931877790833823382854461669921875;0.1545229999999999936921568632897105999290943145751953125;1.0707899999999999085531499076751060783863067626953125;0.0936854000000000020076384998901630751788616180419921875;-2.047680000000000166693325809319503605365753173828125;1.2067900000000000293454149868921376764774322509765625;-0.0189352999999999986602272628033460932783782482147216796875;-0.5821039999999999547952711509424261748790740966796875;-0.80640400000000000968469748841016553342342376708984375;-2.419849999999999834443542567896656692028045654296875;-4.54863999999999979451104081817902624607086181640625;-1.110300000000000064659388954169116914272308349609375;-0.67197099999999998498623199338908307254314422607421875;0.8908110000000000194830818145419470965862274169921875;-1.04140999999999994685140336514450609683990478515625;2.080789999999999917434934104676358401775360107421875;0.802197000000000048913761929725296795368194580078125;0.286883999999999972363440292610903270542621612548828125;-2.20877999999999996560973158921115100383758544921875;-0.5813390000000000501501062899478711187839508056640625;-0.5556680000000000507753838974167592823505401611328125;0.211378000000000010327738664273056201636791229248046875;0.1809360000000000134878774815661017782986164093017578125;0.845323000000000046583181756432168185710906982421875;-2.14989000000000007872813512221910059452056884765625;-0.08759390000000000231494823310640640556812286376953125;-3.036189999999999944435558063560165464878082275390625;-3.2964099999999998402699930011294782161712646484375;0.91354699999999999793232063893810845911502838134765625;-1.791760000000000019326762412674725055694580078125;-0.97237399999999996058619444738724268972873687744140625;1.00208999999999992525090419803746044635772705078125;-0.00607804999999999977233766657036539982073009014129638671875;2.337849999999999983657517077517695724964141845703125;-2.5579499999999999459987520822323858737945556640625;0.345063999999999981849185815008240751922130584716796875]
      mask Display: e = 0.06;c1 = [120 150 230]/255;c2 = ([120 150 230]+0)/383;c3 = ([120 150 230]+128)/383;patch([0 1 1 0],[0 0 1 1],c1);patch([0 1 1 1-e 1-e 0+e],[0 0 1 1-e 0+e 0+e],c2);patch([0 0 1 1-e 0+e 0+e],[0 1 1 1-e 1-e 0+e],c3);plot([0 1 1 0 0],[0 0 1 1 0]);disp('b');
  netsum : Sum  Inputs=2  SaturateOnIntegerOverflow=on  InputSameDT=on  OutDataTypeStr=Inherit: Same as first input
      mask Display: e = 0.06;c1 = [120 150 230]/255;c2 = ([120 150 230]+0)/383;c3 = ([120 150 230]+128)/383;patch([0 1 1 0],[0 0 1 1],c1);patch([0 1 1 1-e 1-e 0+e],[0 0 1 1-e 0+e 0+e],c2);patch([0 0 1 1-e 0+e 0+e],[0 1 1 1-e 1-e 0+e],c3);plot([0 1 1 0 0],[0 0 1 1 0]);disp('+');
  poslin : neural/Transfer Functions/poslin
      settings: SourceProductName=Deep Learning Toolbox  SourceProductBaseCode=NN
  "a{4}" : Outport  InitialOutput=[0;0;0;0;0;0;0;0;0;0;0;0;0;0;0;0;0;0;0;0;0;0;0;0;0;0;0;0;0;0;0;0;0;0;0;0;0;0;0;0;0;0;0;0;0;0;0;0;0;0]
      mask Display: e = 0.06;c1 = [120 230 180]/255;c2 = ([120 230 180]+0)/(256+128);c3 = ([120 230 180]+128)/(256+128);patch([0 1 1 0],[0 0 1 1],c1);patch([0 1 1 1-e 1-e 0+e],[0 0 1 1-e 0+e 0+e],c2);patch([0 0 1 1-e 0+e 0+e],[0 1 1 1-e 1-e 0+e],c3);plot([0 1 1 0 0],[0 0 1 1 0]);
  wires:
  poslin/1 -> "a{4}"/1
  netsum/1 -> poslin/1
  "b{4}"/1 -> netsum/2
  "LW{4,3}"/1("lz{4,3}") -> netsum/1
  Delays 1/1("pd{4,3}") -> "LW{4,3}"/1("ad{4,3}")
  "a{3} "/1 -> Delays 1/1("p{4}")
